simulink model "VirtualADAPT"  (Simulink 8.9)
settings: Solver=ode45  StartTime=0.0  StopTime=inf  RelTol=1e-3  EnableMultiTasking=on
PostLoadFcn:
  | FaultInjectionGUIHandle = FaultInjectionGUI;
InitFcn:
  | VirtualADAPTDataStructure
undefined symbols (not defined in the file or in its folder): ln
libraries: simulink x363
(part 3 of 26)

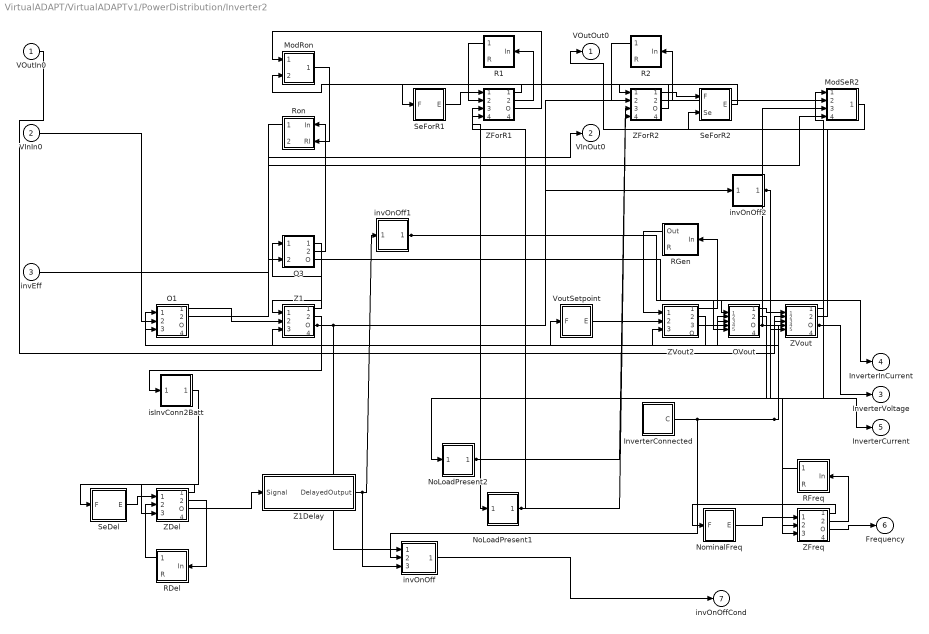
== system VirtualADAPT/VirtualADAPTv1/PowerDistribution/Inverter2 ==
  VOutIn0 : Inport
  VInIn0 : Inport  Port=2
  invEff : Inport  Port=3
  InverterConnected : Subsystem  mask{Constant=1, FP=Nominal, FT=0, FM=0}
      mask prompts: Constant "Value of Constant:"; FP "Fault Profile:"; FT "Fault Injection Time:"; FM "Fault Magnitude/Slope"
      mask TypeOptions FP: Nominal | Abrupt | Incipient
      mask Display: image(imread('Constant.png','BackgroundColor',[1 1 1]))
  ModRon : Subsystem
      mask Display: image(imread('fx.png','BackgroundColor',[1 1 1]))
  ModSeR2 : Subsystem
      mask Display: image(imread('fx.png','BackgroundColor',[1 1 1]))
  NoLoadPresent1 : Subsystem
      mask Display: image(imread('fx.png','BackgroundColor',[1 1 1]))
  NoLoadPresent2 : Subsystem
      mask Display: image(imread('fx.png','BackgroundColor',[1 1 1]))
  NominalFreq : Subsystem  mask{Se=60, FP=Nominal, FT=0, FM=0}
      mask prompts: Se "Value of Se:"; FP "Fault Profile:"; FT "Fault Injection Time:"; FM "Fault Magnitude/Slope/Bias/StuckAtValue"
      mask TypeOptions FP: Nominal | Abrupt | Incipient | Bias | StuckAt
      mask Display: image(imread('Se.png','BackgroundColor',[1 1 1]))
  O1 : Subsystem
      mask Display: image(imread('1.png','BackgroundColor',[1 1 1]))
  O3 : Subsystem
      mask Display: image(imread('1.png','BackgroundColor',[1 1 1]))
  OVout : Subsystem
      mask Display: image(imread('1.png','BackgroundColor',[1 1 1]))
  R1 : Subsystem  mask{R=1, FP=Nominal, FT=0, FM=0}
      mask prompts: R "Value of R:"; FP "Fault Profile:"; FT "Fault Injection Time:"; FM "Fault Magnitude/Slope"
      mask TypeOptions FP: Nominal | Abrupt | Incipient
      mask Display: image(imread('R.png','BackgroundColor',[1 1 1]))
  R2 : Subsystem  mask{R=1, FP=Nominal, FT=0, FM=0}
      mask prompts: R "Value of R:"; FP "Fault Profile:"; FT "Fault Injection Time:"; FM "Fault Magnitude/Slope"
      mask TypeOptions FP: Nominal | Abrupt | Incipient
      mask Display: image(imread('R.png','BackgroundColor',[1 1 1]))
  RDel : Subsystem  mask{R=1, FP=Nominal, FT=0, FM=0}
      mask prompts: R "Value of R:"; FP "Fault Profile:"; FT "Fault Injection Time:"; FM "Fault Magnitude/Slope"
      mask TypeOptions FP: Nominal | Abrupt | Incipient
      mask Display: image(imread('R.png','BackgroundColor',[1 1 1]))
  RFreq : Subsystem  mask{R=1, FP=Nominal, FT=0, FM=0}
      mask prompts: R "Value of R:"; FP "Fault Profile:"; FT "Fault Injection Time:"; FM "Fault Magnitude/Slope"
      mask TypeOptions FP: Nominal | Abrupt | Incipient
      mask Display: image(imread('R.png','BackgroundColor',[1 1 1]))
  RGen : Subsystem  mask{R=1, FP=Nominal, FT=0, FM=0}
      mask prompts: R "Value of R:"; FP "Fault Profile:"; FT "Fault Injection Time:"; FM "Fault Magnitude/Slope"
      mask TypeOptions FP: Nominal | Abrupt | Incipient
      mask Display: image(imread('R.png','BackgroundColor',[1 1 1]))
  Ron : Subsystem  mask{FP=Nominal, FT=0, FM=0}
      mask prompts: FP "Fault Profile:"; FT "Fault Injection Time:"; FM "Fault Magnitude/Slope"
      mask TypeOptions FP: Nominal | Abrupt | Incipient
      mask Display: image(imread('MR.png','BackgroundColor',[1 1 1]))
  SeDel : Subsystem  mask{Se=1, FP=Nominal, FT=0, FM=0}
      mask prompts: Se "Value of Se:"; FP "Fault Profile:"; FT "Fault Injection Time:"; FM "Fault Magnitude/Slope/Bias/StuckAtValue"
      mask TypeOptions FP: Nominal | Abrupt | Incipient | Bias | StuckAt
      mask Display: image(imread('Se.png','BackgroundColor',[1 1 1]))
  SeForR1 : Subsystem  mask{Se=23, FP=Nominal, FT=0, FM=0}
      mask prompts: Se "Value of Se:"; FP "Fault Profile:"; FT "Fault Injection Time:"; FM "Fault Magnitude/Slope/Bias/StuckAtValue"
      mask TypeOptions FP: Nominal | Abrupt | Incipient | Bias | StuckAt
      mask Display: image(imread('Se.png','BackgroundColor',[1 1 1]))
  SeForR2 : Subsystem  mask{FP=Nominal, FT=0, FM=0}
      mask prompts: FP "Fault Profile:"; FT "Fault Injection Time:"; FM "Fault Magnitude/Slope/Bias/StuckAtValue"
      mask TypeOptions FP: Nominal | Abrupt | Incipient | Bias | StuckAt
      mask Display: image(imread('MSe.png','BackgroundColor',[1 1 1]))
  VoutSetpoint : Subsystem  mask{Se=120.5, FP=Nominal, FT=0, FM=0}
      mask prompts: Se "Value of Se:"; FP "Fault Profile:"; FT "Fault Injection Time:"; FM "Fault Magnitude/Slope/Bias/StuckAtValue"
      mask TypeOptions FP: Nominal | Abrupt | Incipient | Bias | StuckAt
      mask Display: image(imread('Se.png','BackgroundColor',[1 1 1]))
  Z1 : Subsystem
      mask Display: image(imread('0.png','BackgroundColor',[1 1 1]))
  Z1Delay : Subsystem
  ZDel : Subsystem
      mask Display: image(imread('0.png','BackgroundColor',[1 1 1]))
  ZForR1 : Subsystem
      mask Display: image(imread('0.png','BackgroundColor',[1 1 1]))
  ZForR2 : Subsystem
      mask Display: image(imread('0.png','BackgroundColor',[1 1 1]))
  ZFreq : Subsystem
      mask Display: image(imread('0.png','BackgroundColor',[1 1 1]))
  ZVout : Subsystem
      mask Display: image(imread('0.png','BackgroundColor',[1 1 1]))
  ZVout2 : Subsystem
      mask Display: image(imread('0.png','BackgroundColor',[1 1 1]))
  invOnOff : Subsystem
      mask Display: image(imread('fx.png','BackgroundColor',[1 1 1]))
  invOnOff1 : Subsystem
      mask Display: image(imread('fx.png','BackgroundColor',[1 1 1]))
  invOnOff2 : Subsystem
      mask Display: image(imread('fx.png','BackgroundColor',[1 1 1]))
  isInvConn2Batt : Subsystem
      mask Display: image(imread('fx.png','BackgroundColor',[1 1 1]))
  VOutOut0 : Outport
  VInOut0 : Outport  Port=2
  InverterVoltage : Outport  Port=3
  InverterInCurrent : Outport  Port=4
  InverterCurrent : Outport  Port=5
  Frequency : Outport  Port=6
  invOnOffCond : Outport  Port=7
  wires:
  ZVout2/1(y(1)) -> RGen/1(In)
  RGen/1(Out) -> ZVout2/1(u(1))
  SeDel/1(E) -> ZDel/1(u(1))
  ZDel/1(y(1)) -> SeDel/1(F)
  ZDel/2(y(2)) -> RDel/1(In)
  RDel/1(Out) -> ZDel/2(u(2))
  VoutSetpoint/1(E) -> ZVout2/2(u(2))
  ZVout2/2(y(2)) -> VoutSetpoint/1(F)
  Z1/1(y(1)) -> O3/1(u(1))
  O3/1(y(1)) -> Z1/1(u(1))
  OVout/1(y(1)) -> ZVout/1(u(1))
  ZVout/1(y(1)) -> OVout/1(u(1))
  O1/1(y(1)) -> Z1/2(u(2))
  Z1/2(y(2)) -> O1/1(u(1))
  ZVout2/3(y(3)) -> OVout/2(u(2))
  OVout/2(y(2)) -> ZVout2/3(u(3))
  SeForR1/1(E) -> ZForR1/1(u(1))
  ZForR1/1(y(1)) -> SeForR1/1(F)
  SeForR2/1(E) -> ZForR2/1(u(1))
  ZForR2/1(y(1)) -> SeForR2/1(F)
  O3/2(y(2)) -> Ron/1(In)
  Ron/1(Out) -> O3/2(u(2))
  ZForR1/2(y(2)) -> R1/1(In)
  R1/1(Out) -> ZForR1/2(u(2))
  ZForR2/2(y(2)) -> R2/1(In)
  R2/1(Out) -> ZForR2/2(u(2))
  NominalFreq/1(E) -> ZFreq/1(u(1))
  ZFreq/1(y(1)) -> NominalFreq/1(F)
  ZFreq/2(y(2)) -> RFreq/1(In)
  RFreq/1(Out) -> ZFreq/2(u(2))
  VInIn0/1 -> O1/2(u(2))
  O1/2(y(2)) -> VInOut0/1
  VOutIn0/1 -> ZVout/2(u(2))
  ZVout/2(y(2)) -> VOutOut0/1
  ModRon/1(y(1)) -> Ron/2(RI)
  ModSeR2/1(y(1)) -> SeForR2/2(Se)
  NoLoadPresent2/1(y(1)) -> ZForR2/3(u(3)), ZForR1/3(u(3))
  InverterConnected/1(C) -> ZVout/3(u(3)), OVout/3(u(3)), O1/3(u(3)), Z1/3(u(3)), invOnOff/2(u(2))
  invOnOff2/1(y(1)) -> ZVout/4(u(4)), OVout/4(u(4))
  invOnOff1/1(y(1)) -> ZFreq/3(u(3)), OVout/5(u(5)), ZVout/5(u(5))
  isInvConn2Batt/1(y(1)) -> ZDel/3(u(3))
  NoLoadPresent1/1(y(1)) -> ZForR1/4(u(4)), ZForR2/4(u(4))
  ZVout/3(O) -> NoLoadPresent2/1(u(1)), ModSeR2/1(u(1)), InverterVoltage/1
  Z1/3(O) -> invOnOff2/1(u(1)), isInvConn2Batt/1(u(1)), ModSeR2/2(u(2)), invOnOff/1(u(1))
  Z1Delay/1(DelayedOutput) -> invOnOff1/1(u(1)), invOnOff/3(u(3))
  OVout/3(O) -> NoLoadPresent1/1(u(1)), ModSeR2/3(u(3)), InverterCurrent/1
  ZForR1/3(O) -> ModRon/1(u(1))
  ZForR2/3(O) -> ModRon/2(u(2))
  O3/3(O) -> InverterInCurrent/1
  ZDel/3(O) -> Z1Delay/1(Signal)
  ZFreq/3(O) -> Frequency/1
  invOnOff/1(y(1)) -> invOnOffCond/1
  invEff/1 -> ModSeR2/4(u(4))

== system VirtualADAPT/VirtualADAPTv1/PowerDistribution/SensorE165 ==
  TrueValue : Inport
  MSe : Subsystem  mask{FP=Nominal, FT=0, FM=0}
      mask prompts: FP "Fault Profile:"; FT "Fault Injection Time:"; FM "Fault Magnitude/Slope/Bias/StuckAtValue"
      mask TypeOptions FP: Nominal | Abrupt | Incipient | Bias | StuckAt
      mask Display: image(imread('MSe.png','BackgroundColor',[1 1 1]))
  R : Subsystem  mask{R=1, FP=Nominal, FT=0, FM=0}
      mask prompts: R "Value of R:"; FP "Fault Profile:"; FT "Fault Injection Time:"; FM "Fault Magnitude/Slope"
      mask TypeOptions FP: Nominal | Abrupt | Incipient
      mask Display: image(imread('R.png','BackgroundColor',[1 1 1]))
  TrueValueMod : Subsystem
      mask Display: image(imread('fx.png','BackgroundColor',[1 1 1]))
  Value : Subsystem
      mask Display: image(imread('0.png','BackgroundColor',[1 1 1]))
  SensedValue : Outport
  wires:
  Value/1(y(1)) -> R/1(In)
  R/1(Out) -> Value/1(u(1))
  MSe/1(E) -> Value/2(u(2))
  Value/2(y(2)) -> MSe/1(F)
  TrueValueMod/1(y(1)) -> MSe/2(Se)
  TrueValue/1 -> TrueValueMod/1(u(1))
  Value/3(O) -> SensedValue/1

== system VirtualADAPT/VirtualADAPTv1/PowerDistribution/SensorE167 ==
  TrueValue : Inport
  MSe : Subsystem  mask{FP=Nominal, FT=0, FM=0}
      mask prompts: FP "Fault Profile:"; FT "Fault Injection Time:"; FM "Fault Magnitude/Slope/Bias/StuckAtValue"
      mask TypeOptions FP: Nominal | Abrupt | Incipient | Bias | StuckAt
      mask Display: image(imread('MSe.png','BackgroundColor',[1 1 1]))
  R : Subsystem  mask{R=1, FP=Nominal, FT=0, FM=0}
      mask prompts: R "Value of R:"; FP "Fault Profile:"; FT "Fault Injection Time:"; FM "Fault Magnitude/Slope"
      mask TypeOptions FP: Nominal | Abrupt | Incipient
      mask Display: image(imread('R.png','BackgroundColor',[1 1 1]))
  TrueValueMod : Subsystem
      mask Display: image(imread('fx.png','BackgroundColor',[1 1 1]))
  Value : Subsystem
      mask Display: image(imread('0.png','BackgroundColor',[1 1 1]))
  SensedValue : Outport
  wires:
  MSe/1(E) -> Value/1(u(1))
  Value/1(y(1)) -> MSe/1(F)
  Value/2(y(2)) -> R/1(In)
  R/1(Out) -> Value/2(u(2))
  TrueValueMod/1(y(1)) -> MSe/2(Se)
  TrueValue/1 -> TrueValueMod/1(u(1))
  Value/3(O) -> SensedValue/1

== system VirtualADAPT/VirtualADAPTv1/PowerDistribution/SensorE181 ==
  TrueValue : Inport
  MSe : Subsystem  mask{FP=Nominal, FT=0, FM=0}
      mask prompts: FP "Fault Profile:"; FT "Fault Injection Time:"; FM "Fault Magnitude/Slope/Bias/StuckAtValue"
      mask TypeOptions FP: Nominal | Abrupt | Incipient | Bias | StuckAt
      mask Display: image(imread('MSe.png','BackgroundColor',[1 1 1]))
  R : Subsystem  mask{R=1, FP=Nominal, FT=0, FM=0}
      mask prompts: R "Value of R:"; FP "Fault Profile:"; FT "Fault Injection Time:"; FM "Fault Magnitude/Slope"
      mask TypeOptions FP: Nominal | Abrupt | Incipient
      mask Display: image(imread('R.png','BackgroundColor',[1 1 1]))
  TrueValueMod : Subsystem
      mask Display: image(imread('fx.png','BackgroundColor',[1 1 1]))
  Value : Subsystem
      mask Display: image(imread('0.png','BackgroundColor',[1 1 1]))
  SensedValue : Outport
  wires:
  Value/1(y(1)) -> R/1(In)
  R/1(Out) -> Value/1(u(1))
  MSe/1(E) -> Value/2(u(2))
  Value/2(y(2)) -> MSe/1(F)
  TrueValueMod/1(y(1)) -> MSe/2(Se)
  TrueValue/1 -> TrueValueMod/1(u(1))
  Value/3(O) -> SensedValue/1

== system VirtualADAPT/VirtualADAPTv1/PowerDistribution/SensorE265 ==
  TrueValue : Inport
  MSe : Subsystem  mask{FP=Nominal, FT=0, FM=0}
      mask prompts: FP "Fault Profile:"; FT "Fault Injection Time:"; FM "Fault Magnitude/Slope/Bias/StuckAtValue"
      mask TypeOptions FP: Nominal | Abrupt | Incipient | Bias | StuckAt
      mask Display: image(imread('MSe.png','BackgroundColor',[1 1 1]))
  R : Subsystem  mask{R=1, FP=Nominal, FT=0, FM=0}
      mask prompts: R "Value of R:"; FP "Fault Profile:"; FT "Fault Injection Time:"; FM "Fault Magnitude/Slope"
      mask TypeOptions FP: Nominal | Abrupt | Incipient
      mask Display: image(imread('R.png','BackgroundColor',[1 1 1]))
  TrueValueMod : Subsystem
      mask Display: image(imread('fx.png','BackgroundColor',[1 1 1]))
  Value : Subsystem
      mask Display: image(imread('0.png','BackgroundColor',[1 1 1]))
  SensedValue : Outport
  wires:
  MSe/1(E) -> Value/1(u(1))
  Value/1(y(1)) -> MSe/1(F)
  Value/2(y(2)) -> R/1(In)
  R/1(Out) -> Value/2(u(2))
  TrueValueMod/1(y(1)) -> MSe/2(Se)
  TrueValue/1 -> TrueValueMod/1(u(1))
  Value/3(O) -> SensedValue/1

== system VirtualADAPT/VirtualADAPTv1/PowerDistribution/SensorE267 ==
  TrueValue : Inport
  MSe : Subsystem  mask{FP=Nominal, FT=0, FM=0}
      mask prompts: FP "Fault Profile:"; FT "Fault Injection Time:"; FM "Fault Magnitude/Slope/Bias/StuckAtValue"
      mask TypeOptions FP: Nominal | Abrupt | Incipient | Bias | StuckAt
      mask Display: image(imread('MSe.png','BackgroundColor',[1 1 1]))
  R : Subsystem  mask{R=1, FP=Nominal, FT=0, FM=0}
      mask prompts: R "Value of R:"; FP "Fault Profile:"; FT "Fault Injection Time:"; FM "Fault Magnitude/Slope"
      mask TypeOptions FP: Nominal | Abrupt | Incipient
      mask Display: image(imread('R.png','BackgroundColor',[1 1 1]))
  TrueValueMod : Subsystem
      mask Display: image(imread('fx.png','BackgroundColor',[1 1 1]))
  Value : Subsystem
      mask Display: image(imread('0.png','BackgroundColor',[1 1 1]))
  SensedValue : Outport
  wires:
  Value/1(y(1)) -> R/1(In)
  R/1(Out) -> Value/1(u(1))
  MSe/1(E) -> Value/2(u(2))
  Value/2(y(2)) -> MSe/1(F)
  TrueValueMod/1(y(1)) -> MSe/2(Se)
  TrueValue/1 -> TrueValueMod/1(u(1))
  Value/3(O) -> SensedValue/1

== system VirtualADAPT/VirtualADAPTv1/PowerDistribution/SensorE281 ==
  TrueValue : Inport
  MSe : Subsystem  mask{FP=Nominal, FT=0, FM=0}
      mask prompts: FP "Fault Profile:"; FT "Fault Injection Time:"; FM "Fault Magnitude/Slope/Bias/StuckAtValue"
      mask TypeOptions FP: Nominal | Abrupt | Incipient | Bias | StuckAt
      mask Display: image(imread('MSe.png','BackgroundColor',[1 1 1]))
  R : Subsystem  mask{R=1, FP=Nominal, FT=0, FM=0}
      mask prompts: R "Value of R:"; FP "Fault Profile:"; FT "Fault Injection Time:"; FM "Fault Magnitude/Slope"
      mask TypeOptions FP: Nominal | Abrupt | Incipient
      mask Display: image(imread('R.png','BackgroundColor',[1 1 1]))
  TrueValueMod : Subsystem
      mask Display: image(imread('fx.png','BackgroundColor',[1 1 1]))
  Value : Subsystem
      mask Display: image(imread('0.png','BackgroundColor',[1 1 1]))
  SensedValue : Outport
  wires:
  MSe/1(E) -> Value/1(u(1))
  Value/1(y(1)) -> MSe/1(F)
  Value/2(y(2)) -> R/1(In)
  R/1(Out) -> Value/2(u(2))
  TrueValueMod/1(y(1)) -> MSe/2(Se)
  TrueValue/1 -> TrueValueMod/1(u(1))
  Value/3(O) -> SensedValue/1

== system VirtualADAPT/VirtualADAPTv1/PowerDistribution/SensorISH162 ==
  CktBrkOpenSignal : Inport
  Function : Subsystem
      mask Display: image(imread('fx.png','BackgroundColor',[1 1 1]))
  MSe : Subsystem  mask{FP=Nominal, FT=0, FM=0}
      mask prompts: FP "Fault Profile:"; FT "Fault Injection Time:"; FM "Fault Magnitude/Slope/Bias/StuckAtValue"
      mask TypeOptions FP: Nominal | Abrupt | Incipient | Bias | StuckAt
      mask Display: image(imread('MSe.png','BackgroundColor',[1 1 1]))
  R : Subsystem  mask{R=1, FP=Nominal, FT=0, FM=0}
      mask prompts: R "Value of R:"; FP "Fault Profile:"; FT "Fault Injection Time:"; FM "Fault Magnitude/Slope"
      mask TypeOptions FP: Nominal | Abrupt | Incipient
      mask Display: image(imread('R.png','BackgroundColor',[1 1 1]))
  Value : Subsystem
      mask Display: image(imread('0.png','BackgroundColor',[1 1 1]))
  RelayState : Outport
  wires:
  MSe/1(E) -> Value/1(u(1))
  Value/1(y(1)) -> MSe/1(F)
  Value/2(y(2)) -> R/1(In)
  R/1(Out) -> Value/2(u(2))
  Function/1(y(1)) -> MSe/2(Se)
  CktBrkOpenSignal/1 -> Function/1(u(1))
  Value/3(O) -> RelayState/1

== system VirtualADAPT/VirtualADAPTv1/PowerDistribution/SensorISH166 ==
  CktBrkOpenSignal : Inport
  Function : Subsystem
      mask Display: image(imread('fx.png','BackgroundColor',[1 1 1]))
  MSe : Subsystem  mask{FP=Nominal, FT=0, FM=0}
      mask prompts: FP "Fault Profile:"; FT "Fault Injection Time:"; FM "Fault Magnitude/Slope/Bias/StuckAtValue"
      mask TypeOptions FP: Nominal | Abrupt | Incipient | Bias | StuckAt
      mask Display: image(imread('MSe.png','BackgroundColor',[1 1 1]))
  R : Subsystem  mask{R=1, FP=Nominal, FT=0, FM=0}
      mask prompts: R "Value of R:"; FP "Fault Profile:"; FT "Fault Injection Time:"; FM "Fault Magnitude/Slope"
      mask TypeOptions FP: Nominal | Abrupt | Incipient
      mask Display: image(imread('R.png','BackgroundColor',[1 1 1]))
  Value : Subsystem
      mask Display: image(imread('0.png','BackgroundColor',[1 1 1]))
  RelayState : Outport
  wires:
  MSe/1(E) -> Value/1(u(1))
  Value/1(y(1)) -> MSe/1(F)
  Value/2(y(2)) -> R/1(In)
  R/1(Out) -> Value/2(u(2))
  Function/1(y(1)) -> MSe/2(Se)
  CktBrkOpenSignal/1 -> Function/1(u(1))
  Value/3(O) -> RelayState/1

== system VirtualADAPT/VirtualADAPTv1/PowerDistribution/SensorISH180 ==
  CktBrkOpenSignal : Inport
  Function : Subsystem
      mask Display: image(imread('fx.png','BackgroundColor',[1 1 1]))
  MSe : Subsystem  mask{FP=Nominal, FT=0, FM=0}
      mask prompts: FP "Fault Profile:"; FT "Fault Injection Time:"; FM "Fault Magnitude/Slope/Bias/StuckAtValue"
      mask TypeOptions FP: Nominal | Abrupt | Incipient | Bias | StuckAt
      mask Display: image(imread('MSe.png','BackgroundColor',[1 1 1]))
  R : Subsystem  mask{R=1, FP=Nominal, FT=0, FM=0}
      mask prompts: R "Value of R:"; FP "Fault Profile:"; FT "Fault Injection Time:"; FM "Fault Magnitude/Slope"
      mask TypeOptions FP: Nominal | Abrupt | Incipient
      mask Display: image(imread('R.png','BackgroundColor',[1 1 1]))
  Value : Subsystem
      mask Display: image(imread('0.png','BackgroundColor',[1 1 1]))
  RelayState : Outport
  wires:
  Value/1(y(1)) -> R/1(In)
  R/1(Out) -> Value/1(u(1))
  MSe/1(E) -> Value/2(u(2))
  Value/2(y(2)) -> MSe/1(F)
  Function/1(y(1)) -> MSe/2(Se)
  CktBrkOpenSignal/1 -> Function/1(u(1))
  Value/3(O) -> RelayState/1

== system VirtualADAPT/VirtualADAPTv1/PowerDistribution/SensorISH262 ==
  CktBrkOpenSignal : Inport
  Function : Subsystem
      mask Display: image(imread('fx.png','BackgroundColor',[1 1 1]))
  MSe : Subsystem  mask{FP=Nominal, FT=0, FM=0}
      mask prompts: FP "Fault Profile:"; FT "Fault Injection Time:"; FM "Fault Magnitude/Slope/Bias/StuckAtValue"
      mask TypeOptions FP: Nominal | Abrupt | Incipient | Bias | StuckAt
      mask Display: image(imread('MSe.png','BackgroundColor',[1 1 1]))
  R : Subsystem  mask{R=1, FP=Nominal, FT=0, FM=0}
      mask prompts: R "Value of R:"; FP "Fault Profile:"; FT "Fault Injection Time:"; FM "Fault Magnitude/Slope"
      mask TypeOptions FP: Nominal | Abrupt | Incipient
      mask Display: image(imread('R.png','BackgroundColor',[1 1 1]))
  Value : Subsystem
      mask Display: image(imread('0.png','BackgroundColor',[1 1 1]))
  RelayState : Outport
  wires:
  Value/1(y(1)) -> R/1(In)
  R/1(Out) -> Value/1(u(1))
  MSe/1(E) -> Value/2(u(2))
  Value/2(y(2)) -> MSe/1(F)
  Function/1(y(1)) -> MSe/2(Se)
  CktBrkOpenSignal/1 -> Function/1(u(1))
  Value/3(O) -> RelayState/1

== system VirtualADAPT/VirtualADAPTv1/PowerDistribution/SensorISH266 ==
  CktBrkOpenSignal : Inport
  Function : Subsystem
      mask Display: image(imread('fx.png','BackgroundColor',[1 1 1]))
  MSe : Subsystem  mask{FP=Nominal, FT=0, FM=0}
      mask prompts: FP "Fault Profile:"; FT "Fault Injection Time:"; FM "Fault Magnitude/Slope/Bias/StuckAtValue"
      mask TypeOptions FP: Nominal | Abrupt | Incipient | Bias | StuckAt
      mask Display: image(imread('MSe.png','BackgroundColor',[1 1 1]))
  R : Subsystem  mask{R=1, FP=Nominal, FT=0, FM=0}
      mask prompts: R "Value of R:"; FP "Fault Profile:"; FT "Fault Injection Time:"; FM "Fault Magnitude/Slope"
      mask TypeOptions FP: Nominal | Abrupt | Incipient
      mask Display: image(imread('R.png','BackgroundColor',[1 1 1]))
  Value : Subsystem
      mask Display: image(imread('0.png','BackgroundColor',[1 1 1]))
  RelayState : Outport
  wires:
  Value/1(y(1)) -> R/1(In)
  R/1(Out) -> Value/1(u(1))
  MSe/1(E) -> Value/2(u(2))
  Value/2(y(2)) -> MSe/1(F)
  Function/1(y(1)) -> MSe/2(Se)
  CktBrkOpenSignal/1 -> Function/1(u(1))
  Value/3(O) -> RelayState/1

== system VirtualADAPT/VirtualADAPTv1/PowerDistribution/SensorISH280 ==
  CktBrkOpenSignal : Inport
  Function : Subsystem
      mask Display: image(imread('fx.png','BackgroundColor',[1 1 1]))
  MSe : Subsystem  mask{FP=Nominal, FT=0, FM=0}
      mask prompts: FP "Fault Profile:"; FT "Fault Injection Time:"; FM "Fault Magnitude/Slope/Bias/StuckAtValue"
      mask TypeOptions FP: Nominal | Abrupt | Incipient | Bias | StuckAt
      mask Display: image(imread('MSe.png','BackgroundColor',[1 1 1]))
  R : Subsystem  mask{R=1, FP=Nominal, FT=0, FM=0}
      mask prompts: R "Value of R:"; FP "Fault Profile:"; FT "Fault Injection Time:"; FM "Fault Magnitude/Slope"
      mask TypeOptions FP: Nominal | Abrupt | Incipient
      mask Display: image(imread('R.png','BackgroundColor',[1 1 1]))
  Value : Subsystem
      mask Display: image(imread('0.png','BackgroundColor',[1 1 1]))
  RelayState : Outport
  wires:
  MSe/1(E) -> Value/1(u(1))
  Value/1(y(1)) -> MSe/1(F)
  Value/2(y(2)) -> R/1(In)
  R/1(Out) -> Value/2(u(2))
  Function/1(y(1)) -> MSe/2(Se)
  CktBrkOpenSignal/1 -> Function/1(u(1))
  Value/3(O) -> RelayState/1

== system VirtualADAPT/VirtualADAPTv1/PowerDistribution/SensorIT167 ==
  TrueValue : Inport
  Offset : Inport  Port=2
  MSe : Subsystem  mask{FP=Nominal, FT=0, FM=0}
      mask prompts: FP "Fault Profile:"; FT "Fault Injection Time:"; FM "Fault Magnitude/Slope/Bias/StuckAtValue"
      mask TypeOptions FP: Nominal | Abrupt | Incipient | Bias | StuckAt
      mask Display: image(imread('MSe.png','BackgroundColor',[1 1 1]))
  Mux : Mux  Inputs=2
  R : Subsystem  mask{R=1, FP=Nominal, FT=0, FM=0}
      mask prompts: R "Value of R:"; FP "Fault Profile:"; FT "Fault Injection Time:"; FM "Fault Magnitude/Slope"
      mask TypeOptions FP: Nominal | Abrupt | Incipient
      mask Display: image(imread('R.png','BackgroundColor',[1 1 1]))
  TrueValueMod : Subsystem
      mask Display: image(imread('fx.png','BackgroundColor',[1 1 1]))
  Value : Subsystem
      mask Display: image(imread('0.png','BackgroundColor',[1 1 1]))
  SensedValue : Outport
  wires:
  MSe/1(E) -> Value/1(u(1))
  Value/1(y(1)) -> MSe/1(F)
  Value/2(y(2)) -> R/1(In)
  R/1(Out) -> Value/2(u(2))
  TrueValueMod/1(y(1)) -> MSe/2(Se)
  Value/3(O) -> SensedValue/1
  TrueValue/1 -> Mux/1
  Offset/1 -> Mux/2
  Mux/1 -> TrueValueMod/1(u(1))

== system VirtualADAPT/VirtualADAPTv1/PowerDistribution/SensorIT181 ==
  TrueValue : Inport
  MSe : Subsystem  mask{FP=Nominal, FT=0, FM=0}
      mask prompts: FP "Fault Profile:"; FT "Fault Injection Time:"; FM "Fault Magnitude/Slope/Bias/StuckAtValue"
      mask TypeOptions FP: Nominal | Abrupt | Incipient | Bias | StuckAt
      mask Display: image(imread('MSe.png','BackgroundColor',[1 1 1]))
  R : Subsystem  mask{R=1, FP=Nominal, FT=0, FM=0}
      mask prompts: R "Value of R:"; FP "Fault Profile:"; FT "Fault Injection Time:"; FM "Fault Magnitude/Slope"
      mask TypeOptions FP: Nominal | Abrupt | Incipient
      mask Display: image(imread('R.png','BackgroundColor',[1 1 1]))
  TrueValueMod : Subsystem
      mask Display: image(imread('fx.png','BackgroundColor',[1 1 1]))
  Value : Subsystem
      mask Display: image(imread('0.png','BackgroundColor',[1 1 1]))
  SensedValue : Outport
  wires:
  MSe/1(E) -> Value/1(u(1))
  Value/1(y(1)) -> MSe/1(F)
  Value/2(y(2)) -> R/1(In)
  R/1(Out) -> Value/2(u(2))
  TrueValueMod/1(y(1)) -> MSe/2(Se)
  TrueValue/1 -> TrueValueMod/1(u(1))
  Value/3(O) -> SensedValue/1

== system VirtualADAPT/VirtualADAPTv1/PowerDistribution/SensorIT267 ==
  TrueValue : Inport
  OffSet : Inport  Port=2
  MSe : Subsystem  mask{FP=Nominal, FT=0, FM=0}
      mask prompts: FP "Fault Profile:"; FT "Fault Injection Time:"; FM "Fault Magnitude/Slope/Bias/StuckAtValue"
      mask TypeOptions FP: Nominal | Abrupt | Incipient | Bias | StuckAt
      mask Display: image(imread('MSe.png','BackgroundColor',[1 1 1]))
  Mux : Mux  Inputs=2
  R : Subsystem  mask{R=1, FP=Nominal, FT=0, FM=0}
      mask prompts: R "Value of R:"; FP "Fault Profile:"; FT "Fault Injection Time:"; FM "Fault Magnitude/Slope"
      mask TypeOptions FP: Nominal | Abrupt | Incipient
      mask Display: image(imread('R.png','BackgroundColor',[1 1 1]))
  TrueValueMod : Subsystem
      mask Display: image(imread('fx.png','BackgroundColor',[1 1 1]))
  Value : Subsystem
      mask Display: image(imread('0.png','BackgroundColor',[1 1 1]))
  SensedValue : Outport
  wires:
  Value/1(y(1)) -> R/1(In)
  R/1(Out) -> Value/1(u(1))
  MSe/1(E) -> Value/2(u(2))
  Value/2(y(2)) -> MSe/1(F)
  TrueValueMod/1(y(1)) -> MSe/2(Se)
  Value/3(O) -> SensedValue/1
  TrueValue/1 -> Mux/1
  Mux/1 -> TrueValueMod/1(u(1))
  OffSet/1 -> Mux/2
